simulink model "wlan_phy_rx_pmd"  (Simulink 7.8)
settings: Solver=VariableStepDiscrete  SolverMode=SingleTasking  StartTime=0.0  StopTime=simtime  RelTol=1e-3
PostLoadFcn:
  | wlan_phy_rx_init
InitFcn:
  | wlan_phy_rx_init
StartFcn:
  | wlan_phy_rx_init
values from neighbouring files (symbols this model uses):
  barker_seq20 = 0.95 * circshift(barker_seq20, [0 4]) ./ max(abs(barker_seq20))    [wlan_phy_rx_init.m]
  bit_scrambler_lfsr_bytes = bi2de(reshape(repmat(scr, 1, 8), 8, 127)', 'left-msb')    [wlan_phy_rx_init.m]
  deinterleave_ROM = [deinterleave_ROM interleave_64QAM zeros(1, 512-length(interleave_64QAM))]    [wlan_phy_rx_init.m]
  longCorr_coef_i = [3*real(LTS_corr)]    [wlan_phy_rx_init.m]
  longCorr_coef_q = [3*imag(LTS_corr)]    [wlan_phy_rx_init.m]
  MAX_CP_LEN = 32    [wlan_phy_rx_init.m]
  MAX_NCBPS = 288    [wlan_phy_rx_init.m]
  MAX_NUM_BYTES = 4096    [wlan_phy_rx_init.m]
  MAX_NUM_SC = 64    [wlan_phy_rx_init.m]
  MAX_NUM_SYMS = 600    [wlan_phy_rx_init.m]
  n_bits_preFFT_sampBuff = ceil(log2(4*MAX_NUM_SC))    [wlan_phy_rx_init.m]
  NCBPS_16QAM = 192    [wlan_phy_rx_init.m]
  NCBPS_64QAM = 288    [wlan_phy_rx_init.m]
  NCBPS_BPSK = 48    [wlan_phy_rx_init.m]
  NCBPS_QPSK = 96    [wlan_phy_rx_init.m]
  NDBPS_16QAM12 = 96    [wlan_phy_rx_init.m]
  NDBPS_16QAM34 = 144    [wlan_phy_rx_init.m]
  NDBPS_64QAM23 = 192    [wlan_phy_rx_init.m]
  NDBPS_64QAM34 = 216    [wlan_phy_rx_init.m]
  NDBPS_BPSK12 = 24    [wlan_phy_rx_init.m]
  NDBPS_BPSK34 = 36    [wlan_phy_rx_init.m]
  NDBPS_QPSK12 = 48    [wlan_phy_rx_init.m]
  NDBPS_QPSK34 = 72    [wlan_phy_rx_init.m]
  paylod_vec_samp_time = 8    [wlan_phy_rx_init.m]
  PHY_MIN_PKT_LEN = 14    [wlan_phy_rx_init.m]
  scr_ind_rev = zeros(1,128)    [wlan_phy_rx_init.m]
undefined symbols (not defined in the file or in its folder): gcbh, xlBlockLoadCallback, xlGetNormalizedPeriod, xlipgetpartsetting
libraries: xbsIndex_r4 x3119, simulink x1, xbsEDKLib_r4 x1
(part 6 of 19)

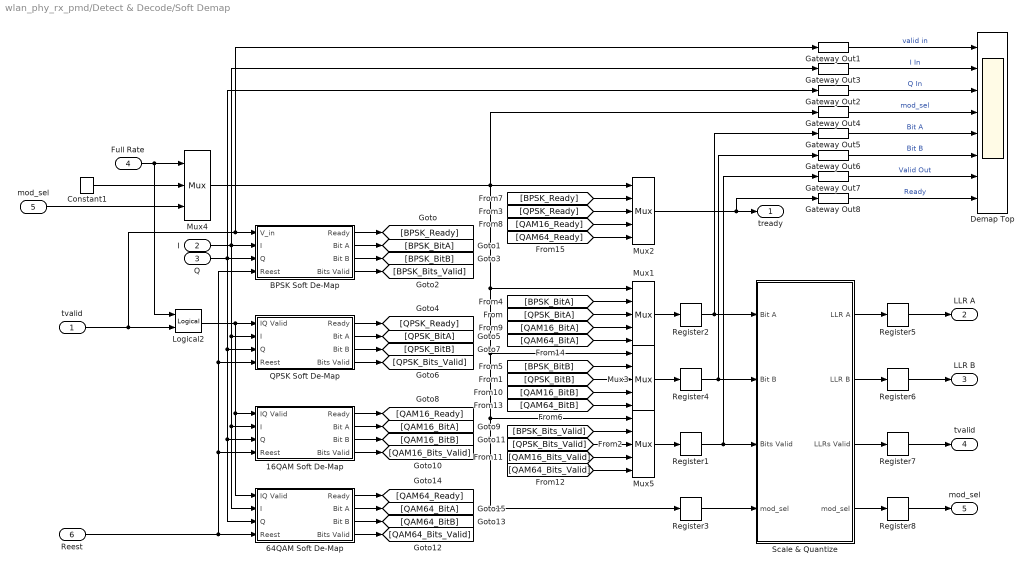
== system wlan_phy_rx_pmd/Detect & Decode/Soft Demap ==
  "tvalid " : Inport
  I : Inport  Port=2
  Q : Inport  Port=3
  Full Rate : Inport  Port=4
  mod_sel : Inport  Port=5
  Reest : Inport  Port=6
  16QAM Soft De-Map : Subsystem
  64QAM Soft De-Map : Subsystem
  BPSK Soft De-Map : Subsystem
  Constant1 : xbsIndex_r4/Constant  const=0  gui_display_data_type=Fixed-point  arith_type=Unsigned  n_bits=2  bin_pt=0  preci_type=Single  exp_width=8  frac_width=24  explicit_period=off  period=1  equ=P=C  opselect=C  inp2=PCIN>>17  opr=+  inp1=P  carry=CIN  dbl_ovrd=off  block_type=constant
      settings: block_version=VER_STRING_GOES_HERE
  Demap Top : Scope  NumInputPorts=8
      settings: TimeRange=20000  YMin=-0.1~-1~0~0~-1~0~-0.1~-1  YMax=1.1~1~1~1~1~1~1.1~1  SaveName=ScopeData5
  Gateway Out{1..8} (8 alike) : xbsIndex_r4/Gateway Out  inherit_from_input=off  hdl_port=off  timing_constraint=None  locs_specified=off  LOCs={}  UseAsDAC=off  DACChannel='1'  block_type=gatewayout
  Logical2 : xbsIndex_r4/Logical  logical_function=AND  inputs=2  en=off  latency=0  precision=Full  arith_type=Unsigned  n_bits=16  bin_pt=0  align_bp=on  dbl_ovrd=off  block_type=logical
  Mux{1..3,5} (4 alike) : xbsIndex_r4/Mux  inputs=4  en=off  latency=0  precision=Full  arith_type=Unsigned  n_bits=16  bin_pt=14  quantization=Truncate  overflow=Wrap  dbl_ovrd=off  block_type=mux
  Mux4 : xbsIndex_r4/Mux  inputs=2  en=off  latency=0  precision=Full  arith_type=Unsigned  n_bits=16  bin_pt=14  quantization=Truncate  overflow=Wrap  dbl_ovrd=off  block_type=mux
  QPSK Soft De-Map : Subsystem
  Register{1..8} (8 alike) : xbsIndex_r4/Register  init=0  rst=off  en=off  dbl_ovrd=off  block_type=register
  Scale & Quantize : Subsystem
  tready : Outport
  LLR A : Outport  Port=2
  LLR B : Outport  Port=3
  tvalid : Outport  Port=4
  " mod_sel" : Outport  Port=5
  wires:
  "tvalid "/1 -> Logical2/2, BPSK Soft De-Map/1(V_in), Gateway Out1/1
  Mux4/1 -> Mux1/1, Mux3/1, Mux5/1, Register3/1, Gateway Out4/1, Mux2/1
  Mux2/1 -> tready/1, Gateway Out8/1
  Gateway Out2/1 -> Demap Top/3  "Q In"
  Gateway Out1/1 -> Demap Top/1  "valid in"
  Gateway Out3/1 -> Demap Top/2  "I In"
  Gateway Out4/1 -> Demap Top/4  "mod_sel"
  mod_sel/1 -> Mux4/3
  Full Rate/1 -> Mux4/1, Logical2/1
  Gateway Out5/1 -> Demap Top/5  "Bit A"
  Gateway Out6/1 -> Demap Top/6  "Bit B"
  Scale & Quantize/1(LLR A) -> Register5/1
  Scale & Quantize/3(LLRs Valid) -> Register7/1
  Scale & Quantize/4(" mod_sel") -> Register8/1
  Gateway Out7/1 -> Demap Top/7  "Valid Out"
  Constant1/1 -> Mux4/2
  Register2/1 -> Gateway Out5/1, Scale & Quantize/1(Bit A)
  Register1/1 -> Scale & Quantize/3(Bits Valid), Gateway Out7/1
  Register3/1 -> Scale & Quantize/4(mod_sel)
  BPSK Soft De-Map/1(Ready) -> [BPSK_Ready]
  BPSK Soft De-Map/2(Bit A) -> [BPSK_BitA]
  BPSK Soft De-Map/3(Bit B) -> [BPSK_BitB]
  BPSK Soft De-Map/4(Bits Valid) -> [BPSK_Bits_Valid]
  QPSK Soft De-Map/1(Ready) -> [QPSK_Ready]
  QPSK Soft De-Map/2(Bit A) -> [QPSK_BitA]
  QPSK Soft De-Map/3(Bit B) -> [QPSK_BitB]
  QPSK Soft De-Map/4(Bits Valid) -> [QPSK_Bits_Valid]
  I/1 -> BPSK Soft De-Map/2(I), Gateway Out3/1, QPSK Soft De-Map/2(I), 16QAM Soft De-Map/2(I), 64QAM Soft De-Map/2(I)
  Q/1 -> BPSK Soft De-Map/3(Q), Gateway Out2/1, QPSK Soft De-Map/3(Q), 16QAM Soft De-Map/3(Q), 64QAM Soft De-Map/3(Q)
  Logical2/1 -> QPSK Soft De-Map/1(IQ Valid), 16QAM Soft De-Map/1(IQ Valid), 64QAM Soft De-Map/1(IQ Valid)
  [BPSK_Ready] -> Mux2/2
  [QPSK_Ready] -> Mux2/3
  Mux1/1 -> Register2/1
  [QPSK_BitA] -> Mux1/3
  [BPSK_BitA] -> Mux1/2
  Register4/1 -> Scale & Quantize/2(Bit B), Gateway Out6/1
  Mux3/1 -> Register4/1
  [BPSK_BitB] -> Mux3/2
  [QPSK_BitB] -> Mux3/3
  Mux5/1 -> Register1/1
  [BPSK_Bits_Valid] -> Mux5/2
  [QPSK_Bits_Valid] -> Mux5/3
  Scale & Quantize/2(LLR B) -> Register6/1
  Gateway Out8/1 -> Demap Top/8  "Ready"
  16QAM Soft De-Map/1(Ready) -> [QAM16_Ready]
  16QAM Soft De-Map/2(Bit A) -> [QAM16_BitA]
  16QAM Soft De-Map/3(Bit B) -> [QAM16_BitB]
  16QAM Soft De-Map/4(Bits Valid) -> [QAM16_Bits_Valid]
  [QAM16_Ready] -> Mux2/4
  [QAM16_BitA] -> Mux1/4
  [QAM16_BitB] -> Mux3/4
  [QAM16_Bits_Valid] -> Mux5/4
  64QAM Soft De-Map/1(Ready) -> [QAM64_Ready]
  64QAM Soft De-Map/2(Bit A) -> [QAM64_BitA]
  64QAM Soft De-Map/3(Bit B) -> [QAM64_BitB]
  64QAM Soft De-Map/4(Bits Valid) -> [QAM64_Bits_Valid]
  [QAM64_Bits_Valid] -> Mux5/5
  [QAM64_BitB] -> Mux3/5
  [QAM64_BitA] -> Mux1/5
  [QAM64_Ready] -> Mux2/5
  Register5/1 -> LLR A/1
  Register6/1 -> LLR B/1
  Register7/1 -> tvalid/1
  Register8/1 -> " mod_sel"/1
  Reest/1 -> 64QAM Soft De-Map/4(Reest), 16QAM Soft De-Map/4(Reest), QPSK Soft De-Map/4(Reest), BPSK Soft De-Map/4(Reest)

== system wlan_phy_rx_pmd/Detect & Decode/Subcarrier Map ==
  x_valid : Inport
  x_ind : Inport  Port=2
  Constant2 : xbsIndex_r4/Constant  const=MAX_NUM_SC  gui_display_data_type=Fixed-point  arith_type=Unsigned  n_bits=ceil(log2(MAX_NUM_SC))+1  bin_pt=0  preci_type=Single  exp_width=8  frac_width=24  explicit_period=off  period=1  equ=P=C  opselect=C  inp2=PCIN>>17  opr=+  inp1=P  carry=CIN  dbl_ovrd=off  block_type=constant
  Convert : xbsIndex_r4/Convert  gui_display_data_type=Boolean  arith_type=Boolean  n_bits=16  bin_pt=14  float_type=Single  exp_bits=8  fraction_bits=24  quantization=Truncate  overflow=Wrap  en=off  latency=0  dbl_ovrd=off  pipeline=off  block_type=convert
  Logical : xbsIndex_r4/Logical  logical_function=AND  inputs=2  en=off  latency=0  precision=Full  arith_type=Unsigned  n_bits=16  bin_pt=0  align_bp=on  dbl_ovrd=off  block_type=logical
  Relational1 : xbsIndex_r4/Relational  mode=a!=b  en=off  latency=0  dbl_ovrd=off  block_type=relational
  SC Sym Map : xbsIndex_r4/ROM  depth=MAX_NUM_SC  initVector=sc_data_sym_map  distributed_mem=Distributed memory  rst=off  init_reg=0  en=off  latency=0  gui_display_data_type=Fixed-point  arith_type=Unsigned  n_bits=ceil(log2(MAX_NUM_SC))+1  bin_pt=0  preci_type=Single  exp_width=8  frac_width=24  dbl_ovrd=off  optimize=Area  use_rpm=on  block_type=sprom
  data_sym_valid : Outport
  wires:
  x_ind/1 -> SC Sym Map/1
  Constant2/1 -> Relational1/1
  SC Sym Map/1 -> Relational1/2
  Relational1/1 -> Logical/2
  Logical/1 -> data_sym_valid/1
  x_valid/1 -> Convert/1
  Convert/1 -> Logical/1

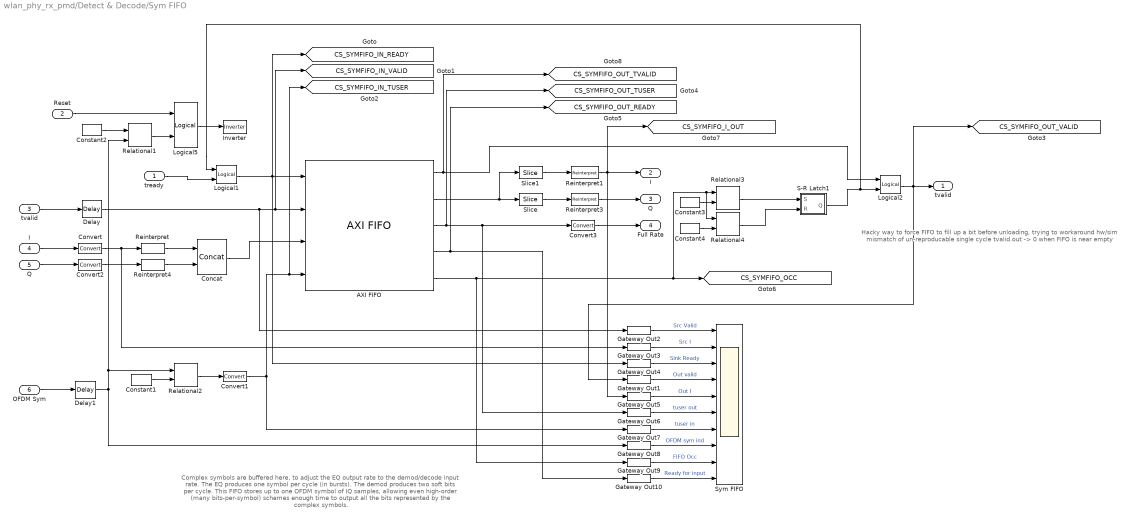
== system wlan_phy_rx_pmd/Detect & Decode/Sym FIFO ==
  note: Complex symbols are buffered here, to adjust the EQ output rate
      | to the demod/decode input rate. The EQ produces one symbol per
      | cycle (in bursts). The demod produces two soft bits per cycle. This
      | FIFO stores up to one OFDM symbol of IQ samples, allowing even
      | high-order (many bits-per-symbol) schemes enough time to output
      | all the bits represented by the complex symbols.
  note: Hacky way to force FIFO to fill up a bit
      | before unloading, trying to workaround
      | hw/sim mismatch of un-reproducable
      | single cycle tvalid.out -> 0 when
      |  FIFO is near empty
  tready : Inport
  Reset : Inport  Port=2
  tvalid : Inport  Port=3
  I : Inport  Port=4
  Q : Inport  Port=5
  OFDM Sym : Inport  Port=6
  AXI FIFO : xbsIndex_r4/AXI FIFO  input_depth_axis=128  actual_fifo_depth_label=130  enable_tdata=on  enable_tdest=off  enable_tstrb=off  enable_tready=on  enable_tid=off  enable_tuser=on  enable_tkeep=off  enable_tlast=off  has_aresetn=off  enable_data_counts_axis=on  fifo_implementation_type_axis=Common Clock Block RAM  trim_axipin_name=on  programmable_full_type_axis=Full  full_threshold_assert_value_axis=0  programmable_empty_type_axis=Empty  empty_threshold_assert_value_axis=1022  block_type=axi_fifo
  Concat : xbsIndex_r4/Concat  num_inputs=2  dbl_ovrd=off  block_type=concat
  Constant1 : xbsIndex_r4/Constant  const=3  gui_display_data_type=Fixed-point  arith_type=Unsigned  n_bits=2  bin_pt=0  preci_type=Single  exp_width=8  frac_width=24  explicit_period=off  period=1  equ=P=C  opselect=C  inp2=PCIN>>17  opr=+  inp1=P  carry=CIN  dbl_ovrd=off  block_type=constant
  Constant2 : xbsIndex_r4/Constant  const=0  gui_display_data_type=Fixed-point  arith_type=Unsigned  n_bits=2  bin_pt=0  preci_type=Single  exp_width=8  frac_width=24  explicit_period=off  period=1  equ=P=C  opselect=C  inp2=PCIN>>17  opr=+  inp1=P  carry=CIN  dbl_ovrd=off  block_type=constant
  Constant3 : xbsIndex_r4/Constant  const=20  gui_display_data_type=Fixed-point  arith_type=Unsigned  n_bits=5  bin_pt=0  preci_type=Single  exp_width=8  frac_width=24  explicit_period=off  period=1  equ=P=C  opselect=C  inp2=PCIN>>17  opr=+  inp1=P  carry=CIN  dbl_ovrd=off  block_type=constant
  Constant4 : xbsIndex_r4/Constant  const=0  gui_display_data_type=Fixed-point  arith_type=Unsigned  n_bits=5  bin_pt=0  preci_type=Single  exp_width=8  frac_width=24  explicit_period=off  period=1  equ=P=C  opselect=C  inp2=PCIN>>17  opr=+  inp1=P  carry=CIN  dbl_ovrd=off  block_type=constant
  Convert : xbsIndex_r4/Convert  gui_display_data_type=Fixed-point  arith_type="Signed  (2's comp)"  n_bits=8  bin_pt=7  float_type=Single  exp_bits=8  fraction_bits=24  quantization=Truncate  overflow=Saturate  en=off  latency=1  dbl_ovrd=off  pipeline=off  block_type=convert
  Convert1 : xbsIndex_r4/Convert  gui_display_data_type=Fixed-point  arith_type=Unsigned  n_bits=1  bin_pt=0  float_type=Single  exp_bits=8  fraction_bits=24  quantization=Truncate  overflow=Saturate  en=off  latency=0  dbl_ovrd=off  pipeline=off  block_type=convert
  Convert2 : xbsIndex_r4/Convert  gui_display_data_type=Fixed-point  arith_type="Signed  (2's comp)"  n_bits=8  bin_pt=7  float_type=Single  exp_bits=8  fraction_bits=24  quantization=Truncate  overflow=Saturate  en=off  latency=1  dbl_ovrd=off  pipeline=off  block_type=convert
  Convert3 : xbsIndex_r4/Convert  gui_display_data_type=Boolean  arith_type=Boolean  n_bits=1  bin_pt=0  float_type=Single  exp_bits=8  fraction_bits=24  quantization=Truncate  overflow=Saturate  en=off  latency=0  dbl_ovrd=off  pipeline=off  block_type=convert
  Delay : xbsIndex_r4/Delay  rst=off  infoeditControl=Selection of Reset will increase slice count due to use of real FFs and instead of SRLs  en=off  latency=1  dbl_ovrd=off  reg_retiming=off  block_type=delay
  Delay1 : xbsIndex_r4/Delay  rst=off  infoeditControl=Selection of Reset will increase slice count due to use of real FFs and instead of SRLs  en=off  latency=1  dbl_ovrd=off  reg_retiming=off  block_type=delay
  Gateway Out{1..10} (10 alike) : xbsIndex_r4/Gateway Out  inherit_from_input=off  hdl_port=off  timing_constraint=None  locs_specified=off  LOCs={}  UseAsDAC=off  DACChannel='1'  block_type=gatewayout
  Inverter : xbsIndex_r4/Inverter  en=off  latency=0  dbl_ovrd=off  block_type=inv
  Logical1 : xbsIndex_r4/Logical  logical_function=AND  inputs=2  en=off  latency=0  precision=Full  arith_type=Unsigned  n_bits=16  bin_pt=0  align_bp=on  dbl_ovrd=off  block_type=logical
  Logical2 : xbsIndex_r4/Logical  logical_function=AND  inputs=2  en=off  latency=0  precision=Full  arith_type=Unsigned  n_bits=16  bin_pt=0  align_bp=on  dbl_ovrd=off  block_type=logical
  Logical5 : xbsIndex_r4/Logical  logical_function=OR  inputs=2  en=off  latency=0  precision=Full  arith_type=Unsigned  n_bits=16  bin_pt=0  align_bp=on  dbl_ovrd=off  block_type=logical
  Reinterpret : xbsIndex_r4/Reinterpret  force_arith_type=on  arith_type=Unsigned  force_bin_pt=on  bin_pt=0  block_type=reinterpret
  Reinterpret1 : xbsIndex_r4/Reinterpret  force_arith_type=on  arith_type="Signed  (2's comp)"  force_bin_pt=on  bin_pt=7  block_type=reinterpret
  Reinterpret3 : xbsIndex_r4/Reinterpret  force_arith_type=on  arith_type="Signed  (2's comp)"  force_bin_pt=on  bin_pt=7  block_type=reinterpret
  Reinterpret4 : xbsIndex_r4/Reinterpret  force_arith_type=on  arith_type=Unsigned  force_bin_pt=on  bin_pt=0  block_type=reinterpret
  Relational1 : xbsIndex_r4/Relational  mode=a=b  en=off  latency=0  dbl_ovrd=off  block_type=relational
  Relational2 : xbsIndex_r4/Relational  mode=a>=b  en=off  latency=0  dbl_ovrd=off  block_type=relational
  Relational3 : xbsIndex_r4/Relational  mode=a>=b  en=off  latency=0  dbl_ovrd=off  block_type=relational
  Relational4 : xbsIndex_r4/Relational  mode=a=b  en=off  latency=0  dbl_ovrd=off  block_type=relational
  S-R Latch1 : Subsystem
  Slice : xbsIndex_r4/Slice  nbits=8  boolean_output=off  mode=Lower Bit Location + Width  bit1=0  base1=MSB of Input  bit0=0  base0=LSB of Input  dbl_ovrd=off  block_type=slice
  Slice1 : xbsIndex_r4/Slice  nbits=8  boolean_output=off  mode=Lower Bit Location + Width  bit1=0  base1=MSB of Input  bit0=8  base0=LSB of Input  dbl_ovrd=off  block_type=slice
  Sym FIFO : Scope  NumInputPorts=10
      settings: TimeRange=20000  YMin=0~-1~0~0~-1~0~0~0~0~-5  YMax=1~1~1~1~1~1~1~5~30~5
  " tvalid" : Outport
  " I" : Outport  Port=2
  " Q" : Outport  Port=3
  Full Rate : Outport  Port=4
  tag scope: CS_SYMFIFO_IN_READY=global, CS_SYMFIFO_IN_TUSER=global, CS_SYMFIFO_IN_VALID=global, CS_SYMFIFO_I_OUT=global, CS_SYMFIFO_OCC=global, CS_SYMFIFO_OUT_READY=global, CS_SYMFIFO_OUT_TUSER=global, CS_SYMFIFO_OUT_TVALID=global, CS_SYMFIFO_OUT_VALID=global
  wires:
  Concat/1 -> AXI FIFO/3
  Slice1/1 -> Reinterpret1/1
  Slice/1 -> Reinterpret3/1
  Logical5/1 -> Inverter/1
  Logical1/1 -> Gateway Out4/1, AXI FIFO/1, [CS_SYMFIFO_IN_READY]
  Reinterpret1/1 -> Gateway Out5/1, " I"/1, [CS_SYMFIFO_I_OUT]
  Reinterpret3/1 -> " Q"/1
  tvalid/1 -> Delay/1
  I/1 -> Convert/1
  Q/1 -> Convert2/1
  Convert/1 -> Reinterpret/1, Gateway Out3/1
  Reinterpret/1 -> Concat/1
  Convert2/1 -> Reinterpret4/1
  Reinterpret4/1 -> Concat/2
  Delay/1 -> Gateway Out2/1, AXI FIFO/2, [CS_SYMFIFO_IN_VALID]
  Gateway Out2/1 -> Sym FIFO/1  "Src Valid"
  Gateway Out3/1 -> Sym FIFO/2  "Src I"
  Gateway Out4/1 -> Sym FIFO/3  "Sink Ready"
  Gateway Out1/1 -> Sym FIFO/4  "Out valid"
  Gateway Out5/1 -> Sym FIFO/5  "Out I"
  Gateway Out6/1 -> Sym FIFO/6  "tuser out"
  Relational2/1 -> Convert1/1
  Constant1/1 -> Relational2/2
  OFDM Sym/1 -> Delay1/1
  AXI FIFO/3 -> Convert3/1, Gateway Out6/1, [CS_SYMFIFO_OUT_TUSER]
  Gateway Out7/1 -> Sym FIFO/7  "tuser in"
  Gateway Out8/1 -> Sym FIFO/8  "OFDM sym ind"
  Convert1/1 -> Gateway Out7/1, AXI FIFO/4, [CS_SYMFIFO_IN_TUSER]
  Convert3/1 -> Full Rate/1
  Delay1/1 -> Gateway Out8/1, Relational2/1, Relational1/2
  AXI FIFO/5 -> Gateway Out9/1, [CS_SYMFIFO_OCC], Relational3/1, Relational4/1
  Gateway Out9/1 -> Sym FIFO/9  "FIFO Occ"
  AXI FIFO/1 -> Logical2/1, [CS_SYMFIFO_OUT_TVALID]
  AXI FIFO/2 -> Slice1/1, Slice/1
  Relational1/1 -> Logical5/2
  Reset/1 -> Logical5/1
  Constant2/1 -> Relational1/1
  AXI FIFO/4 -> Gateway Out10/1, [CS_SYMFIFO_OUT_READY]
  Gateway Out10/1 -> Sym FIFO/10  "Ready for input"
  Logical2/1 -> " tvalid"/1, Gateway Out1/1, [CS_SYMFIFO_OUT_VALID]
  Constant3/1 -> Relational3/2
  Relational3/1 -> S-R Latch1/1(S)
  S-R Latch1/1(Q) -> Logical2/2, Logical1/1
  Constant4/1 -> Relational4/2
  Relational4/1 -> S-R Latch1/2(R)
  tready/1 -> Logical1/2

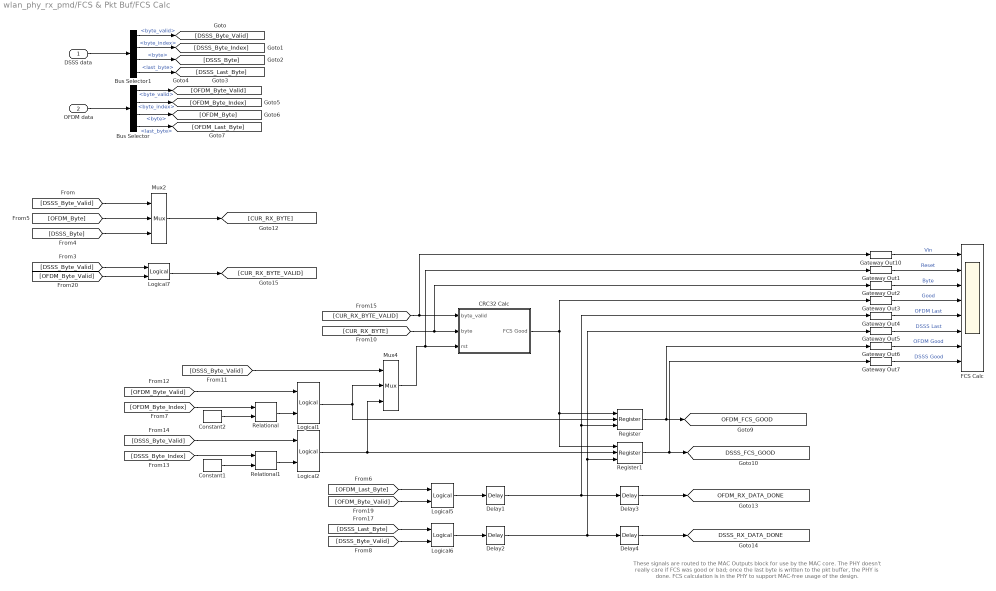
== system wlan_phy_rx_pmd/FCS & Pkt Buf/FCS Calc ==
  note: These signals are routed to the MAC Outputs
      | block for use by the MAC core. The PHY doesn't
      | really care if FCS was good or bad; once the
      | last byte is written to the pkt buffer, the PHY is done.
      | FCS calculation is in the PHY to support MAC-free
      | usage of the design.
  DSSS data : Inport
  OFDM data : Inport  Port=2
  Bus Selector : BusSelector  OutputSignals=byte_valid,byte_index,byte,last_byte
  Bus Selector1 : BusSelector  OutputSignals=byte_valid,byte_index,byte,last_byte
  CRC32 Calc : Subsystem
  Constant1 : xbsIndex_r4/Constant  const=5  gui_display_data_type=Fixed-point  arith_type=Unsigned  n_bits=3  bin_pt=0  preci_type=Single  exp_width=8  frac_width=24  explicit_period=off  period=1  equ=P=C  opselect=C  inp2=PCIN>>17  opr=+  inp1=P  carry=CIN  dbl_ovrd=off  block_type=constant
  Constant2 : xbsIndex_r4/Constant  const=4  gui_display_data_type=Fixed-point  arith_type=Unsigned  n_bits=3  bin_pt=0  preci_type=Single  exp_width=8  frac_width=24  explicit_period=off  period=1  equ=P=C  opselect=C  inp2=PCIN>>17  opr=+  inp1=P  carry=CIN  dbl_ovrd=off  block_type=constant
  Delay{1..4} (4 alike) : xbsIndex_r4/Delay  rst=off  infoeditControl=Selection of Reset will increase slice count due to use of real FFs and instead of SRLs  en=off  latency=1  dbl_ovrd=off  reg_retiming=off  block_type=delay
  FCS Calc : Scope  NumInputPorts=8
      settings: TimeRange=10000  YMin=0~-1~0~-1~-1~-1~-1~-1  YMax=1~1~275~1~1~1~1~1  SaveName=ScopeData22
  Gateway Out{1..7,10} (8 alike) : xbsIndex_r4/Gateway Out  inherit_from_input=off  hdl_port=off  timing_constraint=None  locs_specified=off  LOCs={}  UseAsDAC=off  DACChannel='1'  block_type=gatewayout
  Logical{1..2,5..6} (4 alike) : xbsIndex_r4/Logical  logical_function=AND  inputs=2  en=off  latency=0  precision=Full  arith_type=Unsigned  n_bits=8  bin_pt=2  align_bp=on  dbl_ovrd=off  block_type=logical
      settings: xl_area=[0, 0, 0, 0, 0, 0, 0]  block_version=VER_STRING_GOES_HERE
  Logical7 : xbsIndex_r4/Logical  logical_function=OR  inputs=2  en=off  latency=0  precision=Full  arith_type=Unsigned  n_bits=8  bin_pt=2  align_bp=on  dbl_ovrd=off  block_type=logical
      settings: xl_area=[0, 0, 0, 0, 0, 0, 0]  block_version=VER_STRING_GOES_HERE
  Mux2 : xbsIndex_r4/Mux  inputs=2  en=off  latency=0  precision=Full  arith_type=Unsigned  n_bits=16  bin_pt=14  quantization=Truncate  overflow=Wrap  dbl_ovrd=off  block_type=mux
  Mux4 : xbsIndex_r4/Mux  inputs=2  en=off  latency=0  precision=Full  arith_type=Unsigned  n_bits=16  bin_pt=14  quantization=Truncate  overflow=Wrap  dbl_ovrd=off  block_type=mux
  Register : xbsIndex_r4/Register  init=0  rst=on  en=on  dbl_ovrd=off  block_type=register
  Register1 : xbsIndex_r4/Register  init=0  rst=on  en=on  dbl_ovrd=off  block_type=register
  Relational : xbsIndex_r4/Relational  mode=a<=b  en=off  latency=0  dbl_ovrd=off  block_type=relational
  Relational1 : xbsIndex_r4/Relational  mode=a<=b  en=off  latency=0  dbl_ovrd=off  block_type=relational
  tag scope: DSSS_FCS_GOOD=global, DSSS_RX_DATA_DONE=global, OFDM_FCS_GOOD=global, OFDM_RX_DATA_DONE=global
  wires:
  [DSSS_Byte_Valid] -> Mux2/1
  [OFDM_Byte] -> Mux2/2
  [DSSS_Byte] -> Mux2/3
  Mux2/1 -> [CUR_RX_BYTE]
  [DSSS_Byte_Valid] -> Logical7/1
  [OFDM_Byte_Valid] -> Logical7/2
  Logical7/1 -> [CUR_RX_BYTE_VALID]
  Mux4/1 -> CRC32 Calc/3(rst), Gateway Out1/1
  [DSSS_Byte_Valid] -> Mux4/1
  Register1/1 -> [DSSS_FCS_GOOD], Gateway Out7/1
  Register/1 -> [OFDM_FCS_GOOD], Gateway Out6/1
  CRC32 Calc/1(FCS Good) -> Register/1, Register1/1, Gateway Out3/1
  Delay2/1 -> Delay4/1, Register1/3, Gateway Out5/1
  Delay1/1 -> Delay3/1, Register/3, Gateway Out4/1
  Logical6/1 -> Delay2/1
  Logical5/1 -> Delay1/1
  [DSSS_Byte_Valid] -> Logical6/2
  [DSSS_Last_Byte] -> Logical6/1
  [OFDM_Byte_Valid] -> Logical5/2
  [OFDM_Last_Byte] -> Logical5/1
  [CUR_RX_BYTE_VALID] -> CRC32 Calc/1(byte_valid), Gateway Out10/1
  Logical2/1 -> Mux4/3, Register1/2
  [DSSS_Byte_Valid] -> Logical2/1
  Relational1/1 -> Logical2/2
  Constant1/1 -> Relational1/2
  [DSSS_Byte_Index] -> Relational1/1
  Logical1/1 -> Mux4/2, Register/2
  [OFDM_Byte_Valid] -> Logical1/1
  Relational/1 -> Logical1/2
  Constant2/1 -> Relational/2
  [OFDM_Byte_Index] -> Relational/1
  [CUR_RX_BYTE] -> CRC32 Calc/2(byte), Gateway Out2/1
  Delay3/1 -> [OFDM_RX_DATA_DONE]
  Delay4/1 -> [DSSS_RX_DATA_DONE]
  Gateway Out10/1 -> FCS Calc/1  "Vin"
  Gateway Out1/1 -> FCS Calc/2  "Reset"
  Gateway Out2/1 -> FCS Calc/3  "Byte"
  Gateway Out3/1 -> FCS Calc/4  "Good"
  Gateway Out4/1 -> FCS Calc/5  "OFDM Last"
  Gateway Out5/1 -> FCS Calc/6  "DSSS Last"
  Gateway Out6/1 -> FCS Calc/7  "OFDM Good"
  Gateway Out7/1 -> FCS Calc/8  "DSSS Good"
  Bus Selector1/1 -> [DSSS_Byte_Valid]  "<byte_valid>"
  Bus Selector1/2 -> [DSSS_Byte_Index]  "<byte_index>"
  Bus Selector1/3 -> [DSSS_Byte]  "<byte>"
  Bus Selector1/4 -> [DSSS_Last_Byte]  "<last_byte>"
  Bus Selector/1 -> [OFDM_Byte_Valid]  "<byte_valid>"
  Bus Selector/2 -> [OFDM_Byte_Index]  "<byte_index>"
  Bus Selector/3 -> [OFDM_Byte]  "<byte>"
  Bus Selector/4 -> [OFDM_Last_Byte]  "<last_byte>"
  DSSS data/1 -> Bus Selector1/1
  OFDM data/1 -> Bus Selector/1
